simulink library "inp"  (Simulink 8.2)
settings: Solver=ode45  SolverMode=SingleTasking  StartTime=0.0  StopTime=10.0  RelTol=1e-3
undefined symbols (not defined in the file or in its folder): C_TICK_TIME_S, ENUM_FAULTSTATE_FAIL, ENUM_FAULTSTATE_INDETERMINATE, ENUM_FAULTSTATE_PASS, ENUM_KEYSWITCH_ON, FALSE, KINP_ABS1CANCommuOk_flg_ovrdflg, KINP_ABS1CANCommuOk_flg_ovrdval, KINP_ABS1CANPeriodTime_s, KINP_ABS1CANRolCntOk_flg_ovrdflg, KINP_ABS1CANRolCntOk_flg_ovrdval, KINP_ABS2CANChkSumOk_flg_ovrdflg, KINP_ABS2CANChkSumOk_flg_ovrdval, KINP_ABS2CANCommuOk_flg_ovrdflg, KINP_ABS2CANCommuOk_flg_ovrdval, KINP_ABS2CANPeriodTime_s, KINP_ABS2CANRolCntOk_flg_ovrdflg, KINP_ABS2CANRolCntOk_flg_ovrdval, KINP_ABS3CANCommuOk_flg_ovrdflg, KINP_ABS3CANCommuOk_flg_ovrdval, KINP_ABS4CANCommuOk_flg_ovrdflg, KINP_ABS4CANCommuOk_flg_ovrdval, KINP_ABSActive_flg_defval, KINP_ABSActive_flg_invalid, KINP_ABSActive_flg_ovrdflg, KINP_ABSActive_flg_ovrdval, KINP_ABSActive_flg_setvalid, KINP_ABSActive_flg_srvcflg, KINP_ABSActive_flg_srvcval, KINP_ABSCANAlive_flg_ovrdflg, KINP_ABSCANAlive_flg_ovrdval, KINP_ABSCANCommuOk_flg_ovrdflg, KINP_ABSCANCommuOk_flg_ovrdval, KINP_ABSCANValidOk_flg_ovrdflg, KINP_ABSCANValidOk_flg_ovrdval, KINP_ABSFault_flg_defval, KINP_ABSFault_flg_invalid, KINP_ABSFault_flg_ovrdflg, KINP_ABSFault_flg_ovrdval, KINP_ABSFault_flg_setvalid, KINP_ABSFault_flg_srvcflg, KINP_ABSFault_flg_srvcval, KINP_AC1CANCommuOk_flg_ovrdflg, KINP_AC1CANCommuOk_flg_ovrdval, KINP_ACCANAlive_flg_ovrdflg, KINP_ACCANAlive_flg_ovrdval, KINP_ACCANCommuOk_flg_ovrdflg, KINP_ACCANCommuOk_flg_ovrdval, KINP_ACCANValidOk_flg_ovrdflg, KINP_ACCANValidOk_flg_ovrdval, KINP_ACCCancelH_flg_defval, KINP_ACCCancelH_flg_invalid, KINP_ACCCancelH_flg_ovrdflg, KINP_ACCCancelH_flg_ovrdval, KINP_ACCCancelH_flg_setvalid, KINP_ACCCancelH_flg_srvcflg, KINP_ACCCancelH_flg_srvcval, KINP_AccelPedalPosn_pct_defval, KINP_AccelPedalPosn_pct_gain, KINP_AccelPedalPosn_pct_invalid ... (+1866)
libraries: inp x192, hcu_lib x34, simulink x33
(part 16 of 21)

== system inp/INP/VCU_signals/AckCurPTTqReqApplable ==
  signal_in : Inport
  msg_valid : Inport  Port=2
  Active_fault_flg : Inport  Port=3
  can_signal_process_wio_validity : inp/can_signal_process_wio_validity
  defval : Constant  Value=KINP_AckCurPTTqReqApplable_flg_defval
  invalid : Constant  Value=KINP_AckCurPTTqReqApplable_flg_invalid
  ovrdflg : Constant  Value=KINP_AckCurPTTqReqApplable_flg_ovrdflg
  ovrdval : Constant  Value=KINP_AckCurPTTqReqApplable_flg_ovrdval
  setvalid : Constant  Value=KINP_AckCurPTTqReqApplable_flg_setvalid
  srvcflg : Constant  Value=KINP_AckCurPTTqReqApplable_flg_srvcflg
  srvcval : Constant  Value=KINP_AckCurPTTqReqApplable_flg_srvcval
  signal_out : Outport
  signal_valid_out : Outport  Port=2
  wires:
  can_signal_process_wio_validity/1 -> signal_out/1
  can_signal_process_wio_validity/2 -> signal_valid_out/1
  signal_in/1 -> can_signal_process_wio_validity/1
  msg_valid/1 -> can_signal_process_wio_validity/2
  defval/1 -> can_signal_process_wio_validity/4
  invalid/1 -> can_signal_process_wio_validity/5
  setvalid/1 -> can_signal_process_wio_validity/6
  ovrdflg/1 -> can_signal_process_wio_validity/7
  ovrdval/1 -> can_signal_process_wio_validity/8
  srvcflg/1 -> can_signal_process_wio_validity/9
  srvcval/1 -> can_signal_process_wio_validity/10
  Active_fault_flg/1 -> can_signal_process_wio_validity/3

== system inp/INP/VCU_signals/AckCurVehDec ==
  signal_in : Inport
  signal_valid_in : Inport  Port=2
  msg_valid : Inport  Port=3
  Active_fault_flg : Inport  Port=4
  can_signal_process_with_validity : inp/can_signal_process_with_validity
  conv_gain_offset : Subsystem
  defval : Constant  Value=KINP_AckCurVehDec_mps2_defval
  invalid : Constant  Value=KINP_AckCurVehDec_mps2_invalid
  min_max_limit : Subsystem
  ovrdflg : Constant  Value=KINP_AckCurVehDec_mps2_ovrdflg
  ovrdval : Constant  Value=KINP_AckCurVehDec_mps2_ovrdval
  setvalid : Constant  Value=KINP_AckCurVehDec_mps2_setvalid
  srvcflg : Constant  Value=KINP_AckCurVehDec_mps2_srvcflg
  srvcval : Constant  Value=KINP_AckCurVehDec_mps2_srvcval
  signal_out : Outport
  signal_valid_out : Outport  Port=2
  raw_signal_valid_out : Outport  Port=3
  wires:
  can_signal_process_with_validity/1 -> min_max_limit/1(in)
  can_signal_process_with_validity/2 -> signal_valid_out/1
  signal_in/1 -> conv_gain_offset/1(in)
  signal_valid_in/1 -> can_signal_process_with_validity/2
  msg_valid/1 -> can_signal_process_with_validity/3, can_signal_process_with_validity/5
  defval/1 -> can_signal_process_with_validity/6
  invalid/1 -> can_signal_process_with_validity/7
  conv_gain_offset/1(out) -> can_signal_process_with_validity/1
  min_max_limit/1(out) -> signal_out/1
  setvalid/1 -> can_signal_process_with_validity/8
  ovrdflg/1 -> can_signal_process_with_validity/9
  ovrdval/1 -> can_signal_process_with_validity/10
  srvcflg/1 -> can_signal_process_with_validity/11
  srvcval/1 -> can_signal_process_with_validity/12
  Active_fault_flg/1 -> can_signal_process_with_validity/4
  can_signal_process_with_validity/3 -> raw_signal_valid_out/1

== system inp/INP/VCU_signals/CurDriverTqReq ==
  signal_in : Inport
  signal_valid_in : Inport  Port=2
  msg_valid : Inport  Port=3
  Active_fault_flg : Inport  Port=4
  can_signal_process_with_validity : inp/can_signal_process_with_validity
  conv_gain_offset : Subsystem
  defval : Constant  Value=KINP_CurDriverTqReq_Nm_defval
  invalid : Constant  Value=KINP_CurDriverTqReq_Nm_invalid
  min_max_limit : Subsystem
  ovrdflg : Constant  Value=KINP_CurDriverTqReq_Nm_ovrdflg
  ovrdval : Constant  Value=KINP_CurDriverTqReq_Nm_ovrdval
  setvalid : Constant  Value=KINP_CurDriverTqReq_Nm_setvalid
  srvcflg : Constant  Value=KINP_CurDriverTqReq_Nm_srvcflg
  srvcval : Constant  Value=KINP_CurDriverTqReq_Nm_srvcval
  signal_out : Outport
  signal_valid_out : Outport  Port=2
  raw_signal_valid_out : Outport  Port=3
  wires:
  can_signal_process_with_validity/3 -> raw_signal_valid_out/1
  Active_fault_flg/1 -> can_signal_process_with_validity/4
  srvcval/1 -> can_signal_process_with_validity/12
  srvcflg/1 -> can_signal_process_with_validity/11
  ovrdval/1 -> can_signal_process_with_validity/10
  ovrdflg/1 -> can_signal_process_with_validity/9
  setvalid/1 -> can_signal_process_with_validity/8
  min_max_limit/1(out) -> signal_out/1
  conv_gain_offset/1(out) -> can_signal_process_with_validity/1
  invalid/1 -> can_signal_process_with_validity/7
  defval/1 -> can_signal_process_with_validity/6
  msg_valid/1 -> can_signal_process_with_validity/5, can_signal_process_with_validity/3
  signal_valid_in/1 -> can_signal_process_with_validity/2
  signal_in/1 -> conv_gain_offset/1(in)
  can_signal_process_with_validity/2 -> signal_valid_out/1
  can_signal_process_with_validity/1 -> min_max_limit/1(in)

== system inp/INP/VCU_signals/DeceContManag ==
  signal_in : Inport
  msg_valid : Inport  Port=2
  Active_fault_flg : Inport  Port=3
  can_signal_process_wio_validity : inp/can_signal_process_wio_validity
  defval : Constant  Value=KINP_DeceContManag_flg_defval
  invalid : Constant  Value=KINP_DeceContManag_flg_invalid
  ovrdflg : Constant  Value=KINP_DeceContManag_flg_ovrdflg
  ovrdval : Constant  Value=KINP_DeceContManag_flg_ovrdval
  setvalid : Constant  Value=KINP_DeceContManag_flg_setvalid
  srvcflg : Constant  Value=KINP_DeceContManag_flg_srvcflg
  srvcval : Constant  Value=KINP_DeceContManag_flg_srvcval
  signal_out : Outport
  signal_valid_out : Outport  Port=2
  wires:
  can_signal_process_wio_validity/1 -> signal_out/1
  can_signal_process_wio_validity/2 -> signal_valid_out/1
  signal_in/1 -> can_signal_process_wio_validity/1
  msg_valid/1 -> can_signal_process_wio_validity/2
  defval/1 -> can_signal_process_wio_validity/4
  invalid/1 -> can_signal_process_wio_validity/5
  setvalid/1 -> can_signal_process_wio_validity/6
  ovrdflg/1 -> can_signal_process_wio_validity/7
  ovrdval/1 -> can_signal_process_wio_validity/8
  srvcflg/1 -> can_signal_process_wio_validity/9
  srvcval/1 -> can_signal_process_wio_validity/10
  Active_fault_flg/1 -> can_signal_process_wio_validity/3

== system inp/INP/VCU_signals/DriverBrakPedalOn ==
  signal_in : Inport
  signal_valid_in : Inport  Port=2
  msg_valid : Inport  Port=3
  Active_fault_flg : Inport  Port=4
  can_signal_process_with_validity : inp/can_signal_process_with_validity
  defval : Constant  Value=KINP_DriverBrakPedalOn_flg_defval
  invalid : Constant  Value=KINP_DriverBrakPedalOn_flg_invalid
  ovrdflg : Constant  Value=KINP_DriverBrakPedalOn_flg_ovrdflg
  ovrdval : Constant  Value=KINP_DriverBrakPedalOn_flg_ovrdval
  setvalid : Constant  Value=KINP_DriverBrakPedalOn_flg_setvalid
  srvcflg : Constant  Value=KINP_DriverBrakPedalOn_flg_srvcflg
  srvcval : Constant  Value=KINP_DriverBrakPedalOn_flg_srvcval
  signal_out : Outport
  signal_valid_out : Outport  Port=2
  raw_signal_valid_out : Outport  Port=3
  wires:
  Active_fault_flg/1 -> can_signal_process_with_validity/4
  srvcval/1 -> can_signal_process_with_validity/12
  srvcflg/1 -> can_signal_process_with_validity/11
  ovrdval/1 -> can_signal_process_with_validity/10
  ovrdflg/1 -> can_signal_process_with_validity/9
  setvalid/1 -> can_signal_process_with_validity/8
  invalid/1 -> can_signal_process_with_validity/7
  defval/1 -> can_signal_process_with_validity/6
  msg_valid/1 -> can_signal_process_with_validity/3, can_signal_process_with_validity/5
  signal_valid_in/1 -> can_signal_process_with_validity/2
  signal_in/1 -> can_signal_process_with_validity/1
  can_signal_process_with_validity/2 -> signal_valid_out/1
  can_signal_process_with_validity/1 -> signal_out/1
  can_signal_process_with_validity/3 -> raw_signal_valid_out/1

== system inp/INP/VCU_signals/ECMExtnTqAvailable ==
  signal_in : Inport
  msg_valid : Inport  Port=2
  Active_fault_flg : Inport  Port=3
  can_signal_process_wio_validity : inp/can_signal_process_wio_validity
  defval : Constant  Value=KINP_ECMExtnTqAvailable_flg_defval
  invalid : Constant  Value=KINP_ECMExtnTqAvailable_flg_invalid
  ovrdflg : Constant  Value=KINP_ECMExtnTqAvailable_flg_ovrdflg
  ovrdval : Constant  Value=KINP_ECMExtnTqAvailable_flg_ovrdval
  setvalid : Constant  Value=KINP_ECMExtnTqAvailable_flg_setvalid
  srvcflg : Constant  Value=KINP_ECMExtnTqAvailable_flg_srvcflg
  srvcval : Constant  Value=KINP_ECMExtnTqAvailable_flg_srvcval
  signal_out : Outport
  signal_valid_out : Outport  Port=2
  wires:
  can_signal_process_wio_validity/1 -> signal_out/1
  can_signal_process_wio_validity/2 -> signal_valid_out/1
  signal_in/1 -> can_signal_process_wio_validity/1
  msg_valid/1 -> can_signal_process_wio_validity/2
  defval/1 -> can_signal_process_wio_validity/4
  invalid/1 -> can_signal_process_wio_validity/5
  setvalid/1 -> can_signal_process_wio_validity/6
  ovrdflg/1 -> can_signal_process_wio_validity/7
  ovrdval/1 -> can_signal_process_wio_validity/8
  srvcflg/1 -> can_signal_process_wio_validity/9
  srvcval/1 -> can_signal_process_wio_validity/10
  Active_fault_flg/1 -> can_signal_process_wio_validity/3

== system inp/INP/VCU_signals/ECMTqSource ==
  signal_in : Inport
  msg_valid : Inport  Port=2
  Active_fault_flg : Inport  Port=3
  can_signal_process_wio_validity : inp/can_signal_process_wio_validity
  defval : Constant  Value=KINP_ECMTqSource_enum_defval
  invalid : Constant  Value=KINP_ECMTqSource_enum_invalid
  ovrdflg : Constant  Value=KINP_ECMTqSource_enum_ovrdflg
  ovrdval : Constant  Value=KINP_ECMTqSource_enum_ovrdval
  setvalid : Constant  Value=KINP_ECMTqSource_enum_setvalid
  srvcflg : Constant  Value=KINP_ECMTqSource_enum_srvcflg
  srvcval : Constant  Value=KINP_ECMTqSource_enum_srvcval
  signal_out : Outport
  signal_valid_out : Outport  Port=2
  wires:
  Active_fault_flg/1 -> can_signal_process_wio_validity/3
  srvcval/1 -> can_signal_process_wio_validity/10
  srvcflg/1 -> can_signal_process_wio_validity/9
  ovrdval/1 -> can_signal_process_wio_validity/8
  ovrdflg/1 -> can_signal_process_wio_validity/7
  setvalid/1 -> can_signal_process_wio_validity/6
  invalid/1 -> can_signal_process_wio_validity/5
  defval/1 -> can_signal_process_wio_validity/4
  msg_valid/1 -> can_signal_process_wio_validity/2
  signal_in/1 -> can_signal_process_wio_validity/1
  can_signal_process_wio_validity/2 -> signal_valid_out/1
  can_signal_process_wio_validity/1 -> signal_out/1

== system inp/INP/VCU_signals/ESCBattVolt ==
  signal_in : Inport
  signal_valid_in : Inport  Port=2
  msg_valid : Inport  Port=3
  Active_fault_flg : Inport  Port=4
  can_signal_process_with_validity : inp/can_signal_process_with_validity
  conv_gain_offset : Subsystem
  defval : Constant  Value=KINP_ESCBattVolt_V_defval
  invalid : Constant  Value=KINP_ESCBattVolt_V_invalid
  min_max_limit : Subsystem
  ovrdflg : Constant  Value=KINP_ESCBattVolt_V_ovrdflg
  ovrdval : Constant  Value=KINP_ESCBattVolt_V_ovrdval
  setvalid : Constant  Value=KINP_ESCBattVolt_V_setvalid
  srvcflg : Constant  Value=KINP_ESCBattVolt_V_srvcflg
  srvcval : Constant  Value=KINP_ESCBattVolt_V_srvcval
  signal_out : Outport
  signal_valid_out : Outport  Port=2
  raw_signal_valid_out : Outport  Port=3
  wires:
  can_signal_process_with_validity/3 -> raw_signal_valid_out/1
  Active_fault_flg/1 -> can_signal_process_with_validity/4
  srvcval/1 -> can_signal_process_with_validity/12
  srvcflg/1 -> can_signal_process_with_validity/11
  ovrdval/1 -> can_signal_process_with_validity/10
  ovrdflg/1 -> can_signal_process_with_validity/9
  setvalid/1 -> can_signal_process_with_validity/8
  min_max_limit/1(out) -> signal_out/1
  conv_gain_offset/1(out) -> can_signal_process_with_validity/1
  invalid/1 -> can_signal_process_with_validity/7
  defval/1 -> can_signal_process_with_validity/6
  msg_valid/1 -> can_signal_process_with_validity/5, can_signal_process_with_validity/3
  signal_valid_in/1 -> can_signal_process_with_validity/2
  signal_in/1 -> conv_gain_offset/1(in)
  can_signal_process_with_validity/2 -> signal_valid_out/1
  can_signal_process_with_validity/1 -> min_max_limit/1(in)

== system inp/INP/VCU_signals/ESCBrakeStatus ==
  signal_in : Inport
  msg_valid : Inport  Port=2
  Active_fault_flg : Inport  Port=3
  can_signal_process_wio_validity : inp/can_signal_process_wio_validity
  defval : Constant  Value=KINP_ESCBrakeStatus_enum_defval
  invalid : Constant  Value=KINP_ESCBrakeStatus_enum_invalid
  ovrdflg : Constant  Value=KINP_ESCBrakeStatus_enum_ovrdflg
  ovrdval : Constant  Value=KINP_ESCBrakeStatus_enum_ovrdval
  setvalid : Constant  Value=KINP_ESCBrakeStatus_enum_setvalid
  srvcflg : Constant  Value=KINP_ESCBrakeStatus_enum_srvcflg
  srvcval : Constant  Value=KINP_ESCBrakeStatus_enum_srvcval
  signal_out : Outport
  signal_valid_out : Outport  Port=2
  wires:
  can_signal_process_wio_validity/1 -> signal_out/1
  can_signal_process_wio_validity/2 -> signal_valid_out/1
  signal_in/1 -> can_signal_process_wio_validity/1
  msg_valid/1 -> can_signal_process_wio_validity/2
  defval/1 -> can_signal_process_wio_validity/4
  invalid/1 -> can_signal_process_wio_validity/5
  setvalid/1 -> can_signal_process_wio_validity/6
  ovrdflg/1 -> can_signal_process_wio_validity/7
  ovrdval/1 -> can_signal_process_wio_validity/8
  srvcflg/1 -> can_signal_process_wio_validity/9
  srvcval/1 -> can_signal_process_wio_validity/10
  Active_fault_flg/1 -> can_signal_process_wio_validity/3

== system inp/INP/VCU_signals/ESCExtnBrkAvailable ==
  signal_in : Inport
  msg_valid : Inport  Port=2
  Active_fault_flg : Inport  Port=3
  can_signal_process_wio_validity : inp/can_signal_process_wio_validity
  defval : Constant  Value=KINP_ESCExtnBrkAvailable_flg_defval
  invalid : Constant  Value=KINP_ESCExtnBrkAvailable_flg_invalid
  ovrdflg : Constant  Value=KINP_ESCExtnBrkAvailable_flg_ovrdflg
  ovrdval : Constant  Value=KINP_ESCExtnBrkAvailable_flg_ovrdval
  setvalid : Constant  Value=KINP_ESCExtnBrkAvailable_flg_setvalid
  srvcflg : Constant  Value=KINP_ESCExtnBrkAvailable_flg_srvcflg
  srvcval : Constant  Value=KINP_ESCExtnBrkAvailable_flg_srvcval
  signal_out : Outport
  signal_valid_out : Outport  Port=2
  wires:
  Active_fault_flg/1 -> can_signal_process_wio_validity/3
  srvcval/1 -> can_signal_process_wio_validity/10
  srvcflg/1 -> can_signal_process_wio_validity/9
  ovrdval/1 -> can_signal_process_wio_validity/8
  ovrdflg/1 -> can_signal_process_wio_validity/7
  setvalid/1 -> can_signal_process_wio_validity/6
  invalid/1 -> can_signal_process_wio_validity/5
  defval/1 -> can_signal_process_wio_validity/4
  msg_valid/1 -> can_signal_process_wio_validity/2
  signal_in/1 -> can_signal_process_wio_validity/1
  can_signal_process_wio_validity/2 -> signal_valid_out/1
  can_signal_process_wio_validity/1 -> signal_out/1

== system inp/INP/VCU_signals/HCUMILReq ==
  signal_in : Inport
  msg_valid : Inport  Port=2
  Active_fault_flg : Inport  Port=3
  can_signal_process_wio_validity : inp/can_signal_process_wio_validity
  defval : Constant  Value=KINP_HCUMILReq_flg_defval
  invalid : Constant  Value=KINP_HCUMILReq_flg_invalid
  ovrdflg : Constant  Value=KINP_HCUMILReq_flg_ovrdflg
  ovrdval : Constant  Value=KINP_HCUMILReq_flg_ovrdval
  setvalid : Constant  Value=KINP_HCUMILReq_flg_setvalid
  srvcflg : Constant  Value=KINP_HCUMILReq_flg_srvcflg
  srvcval : Constant  Value=KINP_HCUMILReq_flg_srvcval
  signal_out : Outport
  signal_valid_out : Outport  Port=2
  wires:
  Active_fault_flg/1 -> can_signal_process_wio_validity/3
  srvcval/1 -> can_signal_process_wio_validity/10
  srvcflg/1 -> can_signal_process_wio_validity/9
  ovrdval/1 -> can_signal_process_wio_validity/8
  ovrdflg/1 -> can_signal_process_wio_validity/7
  setvalid/1 -> can_signal_process_wio_validity/6
  invalid/1 -> can_signal_process_wio_validity/5
  defval/1 -> can_signal_process_wio_validity/4
  msg_valid/1 -> can_signal_process_wio_validity/2
  signal_in/1 -> can_signal_process_wio_validity/1
  can_signal_process_wio_validity/2 -> signal_valid_out/1
  can_signal_process_wio_validity/1 -> signal_out/1

== system inp/INP/VCU_signals/HWAccelPedalPosn ==
  signal_in : Inport
  signal_valid_in : Inport  Port=2
  msg_valid : Inport  Port=3
  Active_fault_flg : Inport  Port=4
  can_signal_process_with_validity : inp/can_signal_process_with_validity
  conv_gain_offset : Subsystem
  defval : Constant  Value=KINP_HWAccelPedalPosn_pct_defval
  invalid : Constant  Value=KINP_HWAccelPedalPosn_pct_invalid
  min_max_limit : Subsystem
  ovrdflg : Constant  Value=KINP_HWAccelPedalPosn_pct_ovrdflg
  ovrdval : Constant  Value=KINP_HWAccelPedalPosn_pct_ovrdval
  setvalid : Constant  Value=KINP_HWAccelPedalPosn_pct_setvalid
  srvcflg : Constant  Value=KINP_HWAccelPedalPosn_pct_srvcflg
  srvcval : Constant  Value=KINP_HWAccelPedalPosn_pct_srvcval
  signal_out : Outport
  signal_valid_out : Outport  Port=2
  raw_signal_valid_out : Outport  Port=3
  wires:
  can_signal_process_with_validity/1 -> min_max_limit/1(in)
  can_signal_process_with_validity/2 -> signal_valid_out/1
  signal_in/1 -> conv_gain_offset/1(in)
  signal_valid_in/1 -> can_signal_process_with_validity/2
  msg_valid/1 -> can_signal_process_with_validity/3, can_signal_process_with_validity/5
  defval/1 -> can_signal_process_with_validity/6
  invalid/1 -> can_signal_process_with_validity/7
  conv_gain_offset/1(out) -> can_signal_process_with_validity/1
  min_max_limit/1(out) -> signal_out/1
  setvalid/1 -> can_signal_process_with_validity/8
  ovrdflg/1 -> can_signal_process_with_validity/9
  ovrdval/1 -> can_signal_process_with_validity/10
  srvcflg/1 -> can_signal_process_with_validity/11
  srvcval/1 -> can_signal_process_with_validity/12
  Active_fault_flg/1 -> can_signal_process_with_validity/4
  can_signal_process_with_validity/3 -> raw_signal_valid_out/1

== system inp/INP/VCU_signals/LFCOnVCU ==
  signal_in : Inport
  msg_valid : Inport  Port=2
  Active_fault_flg : Inport  Port=3
  can_signal_process_wio_validity : inp/can_signal_process_wio_validity
  defval : Constant  Value=KINP_LFCOnVCU_flg_defval
  invalid : Constant  Value=KINP_LFCOnVCU_flg_invalid
  ovrdflg : Constant  Value=KINP_LFCOnVCU_flg_ovrdflg
  ovrdval : Constant  Value=KINP_LFCOnVCU_flg_ovrdval
  setvalid : Constant  Value=KINP_LFCOnVCU_flg_setvalid
  srvcflg : Constant  Value=KINP_LFCOnVCU_flg_srvcflg
  srvcval : Constant  Value=KINP_LFCOnVCU_flg_srvcval
  signal_out : Outport
  signal_valid_out : Outport  Port=2
  wires:
  Active_fault_flg/1 -> can_signal_process_wio_validity/3
  srvcval/1 -> can_signal_process_wio_validity/10
  srvcflg/1 -> can_signal_process_wio_validity/9
  ovrdval/1 -> can_signal_process_wio_validity/8
  ovrdflg/1 -> can_signal_process_wio_validity/7
  setvalid/1 -> can_signal_process_wio_validity/6
  invalid/1 -> can_signal_process_wio_validity/5
  defval/1 -> can_signal_process_wio_validity/4
  msg_valid/1 -> can_signal_process_wio_validity/2
  signal_in/1 -> can_signal_process_wio_validity/1
  can_signal_process_wio_validity/2 -> signal_valid_out/1
  can_signal_process_wio_validity/1 -> signal_out/1

== system inp/INP/VCU_signals/MSAOnVCU ==
  signal_in : Inport
  msg_valid : Inport  Port=2
  Active_fault_flg : Inport  Port=3
  can_signal_process_wio_validity : inp/can_signal_process_wio_validity
  defval : Constant  Value=KINP_MSAOnVCU_flg_defval
  invalid : Constant  Value=KINP_MSAOnVCU_flg_invalid
  ovrdflg : Constant  Value=KINP_MSAOnVCU_flg_ovrdflg
  ovrdval : Constant  Value=KINP_MSAOnVCU_flg_ovrdval
  setvalid : Constant  Value=KINP_MSAOnVCU_flg_setvalid
  srvcflg : Constant  Value=KINP_MSAOnVCU_flg_srvcflg
  srvcval : Constant  Value=KINP_MSAOnVCU_flg_srvcval
  signal_out : Outport
  signal_valid_out : Outport  Port=2
  wires:
  can_signal_process_wio_validity/1 -> signal_out/1
  can_signal_process_wio_validity/2 -> signal_valid_out/1
  signal_in/1 -> can_signal_process_wio_validity/1
  msg_valid/1 -> can_signal_process_wio_validity/2
  defval/1 -> can_signal_process_wio_validity/4
  invalid/1 -> can_signal_process_wio_validity/5
  setvalid/1 -> can_signal_process_wio_validity/6
  ovrdflg/1 -> can_signal_process_wio_validity/7
  ovrdval/1 -> can_signal_process_wio_validity/8
  srvcflg/1 -> can_signal_process_wio_validity/9
  srvcval/1 -> can_signal_process_wio_validity/10
  Active_fault_flg/1 -> can_signal_process_wio_validity/3

== system inp/INP/ABS_signals/BrakBoosterPres/conv_gain_offset ==
  in : Inport
  Product : Product  RndMeth=Zero
  Sum : Sum
  gain : Constant  Value=KINP_BrakBoosterPres_kPa_gain
  offset : Constant  Value=KINP_BrakBoosterPres_kPa_offset
  out : Outport
  wires:
  offset/1 -> Sum/2
  Product/1 -> Sum/1
  gain/1 -> Product/2
  in/1 -> Product/1
  Sum/1 -> out/1

== system inp/INP/ABS_signals/BrakBoosterPres/min_max_limit ==
  in : Inport
  MinMax10 : MinMax  Function=max  Inputs=2
  MinMax5 : MinMax  Inputs=2
  maxval : Constant  Value=KINP_BrakBoosterPres_kPa_maxval
  minval : Constant  Value=KINP_BrakBoosterPres_kPa_minval
  out : Outport
  wires:
  minval/1 -> MinMax10/2
  maxval/1 -> MinMax5/2
  MinMax10/1 -> out/1
  in/1 -> MinMax5/1
  MinMax5/1 -> MinMax10/1

== system inp/INP/ABS_signals/LFWheelSpeed/conv_gain_offset ==
  in : Inport
  Product : Product  RndMeth=Zero
  Sum : Sum
  gain : Constant  Value=KINP_LFWheelSpeed_kph_gain
  offset : Constant  Value=KINP_LFWheelSpeed_kph_offset
  out : Outport
  wires:
  offset/1 -> Sum/2
  Product/1 -> Sum/1
  gain/1 -> Product/2
  in/1 -> Product/1
  Sum/1 -> out/1

== system inp/INP/ABS_signals/LFWheelSpeed/min_max_limit ==
  in : Inport
  MinMax10 : MinMax  Function=max  Inputs=2
  MinMax5 : MinMax  Inputs=2
  maxval : Constant  Value=KINP_LFWheelSpeed_kph_maxval
  minval : Constant  Value=KINP_LFWheelSpeed_kph_minval
  out : Outport
  wires:
  minval/1 -> MinMax10/2
  maxval/1 -> MinMax5/2
  MinMax10/1 -> out/1
  in/1 -> MinMax5/1
  MinMax5/1 -> MinMax10/1

== system inp/INP/ABS_signals/LRWheelSpeed/conv_gain_offset ==
  in : Inport
  Product : Product  RndMeth=Zero
  Sum : Sum
  gain : Constant  Value=KINP_LRWheelSpeed_kph_gain
  offset : Constant  Value=KINP_LRWheelSpeed_kph_offset
  out : Outport
  wires:
  Sum/1 -> out/1
  in/1 -> Product/1
  gain/1 -> Product/2
  Product/1 -> Sum/1
  offset/1 -> Sum/2

== system inp/INP/ABS_signals/LRWheelSpeed/min_max_limit ==
  in : Inport
  MinMax10 : MinMax  Function=max  Inputs=2
  MinMax5 : MinMax  Inputs=2
  maxval : Constant  Value=KINP_LRWheelSpeed_kph_maxval
  minval : Constant  Value=KINP_LRWheelSpeed_kph_minval
  out : Outport
  wires:
  MinMax5/1 -> MinMax10/1
  in/1 -> MinMax5/1
  MinMax10/1 -> out/1
  maxval/1 -> MinMax5/2
  minval/1 -> MinMax10/2

== system inp/INP/ABS_signals/LatAccel/conv_gain_offset ==
  in : Inport
  Product : Product  RndMeth=Zero
  Sum : Sum
  gain : Constant  Value=KINP_LatAccel_G_gain
  offset : Constant  Value=KINP_LatAccel_G_offset
  out : Outport
  wires:
  offset/1 -> Sum/2
  Product/1 -> Sum/1
  gain/1 -> Product/2
  in/1 -> Product/1
  Sum/1 -> out/1

== system inp/INP/ABS_signals/LatAccel/min_max_limit ==
  in : Inport
  MinMax10 : MinMax  Function=max  Inputs=2
  MinMax5 : MinMax  Inputs=2
  maxval : Constant  Value=KINP_LatAccel_G_maxval
  minval : Constant  Value=KINP_LatAccel_G_minval
  out : Outport
  wires:
  minval/1 -> MinMax10/2
  maxval/1 -> MinMax5/2
  MinMax10/1 -> out/1
  in/1 -> MinMax5/1
  MinMax5/1 -> MinMax10/1

== system inp/INP/ABS_signals/LongAccel/conv_gain_offset ==
  in : Inport
  Product : Product  RndMeth=Zero
  Sum : Sum
  gain : Constant  Value=KINP_LongAccel_G_gain
  offset : Constant  Value=KINP_LongAccel_G_offset
  out : Outport
  wires:
  Sum/1 -> out/1
  in/1 -> Product/1
  gain/1 -> Product/2
  Product/1 -> Sum/1
  offset/1 -> Sum/2

== system inp/INP/ABS_signals/LongAccel/min_max_limit ==
  in : Inport
  MinMax10 : MinMax  Function=max  Inputs=2
  MinMax5 : MinMax  Inputs=2
  maxval : Constant  Value=KINP_LongAccel_G_maxval
  minval : Constant  Value=KINP_LongAccel_G_minval
  out : Outport
  wires:
  MinMax5/1 -> MinMax10/1
  in/1 -> MinMax5/1
  MinMax10/1 -> out/1
  maxval/1 -> MinMax5/2
  minval/1 -> MinMax10/2

== system inp/INP/ABS_signals/MCPressure/conv_gain_offset ==
  in : Inport
  Product : Product  RndMeth=Zero
  Sum : Sum
  gain : Constant  Value=KINP_MCPressure_bar_gain
  offset : Constant  Value=KINP_MCPressure_bar_offset
  out : Outport
  wires:
  offset/1 -> Sum/2
  Product/1 -> Sum/1
  gain/1 -> Product/2
  in/1 -> Product/1
  Sum/1 -> out/1

== system inp/INP/ABS_signals/MCPressure/min_max_limit ==
  in : Inport
  MinMax10 : MinMax  Function=max  Inputs=2
  MinMax5 : MinMax  Inputs=2
  maxval : Constant  Value=KINP_MCPressure_bar_maxval
  minval : Constant  Value=KINP_MCPressure_bar_minval
  out : Outport
  wires:
  minval/1 -> MinMax10/2
  maxval/1 -> MinMax5/2
  MinMax10/1 -> out/1
  in/1 -> MinMax5/1
  MinMax5/1 -> MinMax10/1

== system inp/INP/ABS_signals/RFWheelSpeed/conv_gain_offset ==
  in : Inport
  Product : Product  RndMeth=Zero
  Sum : Sum
  gain : Constant  Value=KINP_RFWheelSpeed_kph_gain
  offset : Constant  Value=KINP_RFWheelSpeed_kph_offset
  out : Outport
  wires:
  offset/1 -> Sum/2
  Product/1 -> Sum/1
  gain/1 -> Product/2
  in/1 -> Product/1
  Sum/1 -> out/1

== system inp/INP/ABS_signals/RFWheelSpeed/min_max_limit ==
  in : Inport
  MinMax10 : MinMax  Function=max  Inputs=2
  MinMax5 : MinMax  Inputs=2
  maxval : Constant  Value=KINP_RFWheelSpeed_kph_maxval
  minval : Constant  Value=KINP_RFWheelSpeed_kph_minval
  out : Outport
  wires:
  minval/1 -> MinMax10/2
  maxval/1 -> MinMax5/2
  MinMax10/1 -> out/1
  in/1 -> MinMax5/1
  MinMax5/1 -> MinMax10/1

== system inp/INP/ABS_signals/RRWheelSpeed/conv_gain_offset ==
  in : Inport
  Product : Product  RndMeth=Zero
  Sum : Sum
  gain : Constant  Value=KINP_RRWheelSpeed_kph_gain
  offset : Constant  Value=KINP_RRWheelSpeed_kph_offset
  out : Outport
  wires:
  Sum/1 -> out/1
  in/1 -> Product/1
  gain/1 -> Product/2
  Product/1 -> Sum/1
  offset/1 -> Sum/2

== system inp/INP/ABS_signals/RRWheelSpeed/min_max_limit ==
  in : Inport
  MinMax10 : MinMax  Function=max  Inputs=2
  MinMax5 : MinMax  Inputs=2
  maxval : Constant  Value=KINP_RRWheelSpeed_kph_maxval
  minval : Constant  Value=KINP_RRWheelSpeed_kph_minval
  out : Outport
  wires:
  MinMax5/1 -> MinMax10/1
  in/1 -> MinMax5/1
  MinMax10/1 -> out/1
  maxval/1 -> MinMax5/2
  minval/1 -> MinMax10/2

== system inp/INP/ABS_signals/SurfaceGrade/conv_gain_offset ==
  in : Inport
  Product : Product  RndMeth=Zero
  Sum : Sum
  gain : Constant  Value=KINP_SurfaceGrade_deg_gain
  offset : Constant  Value=KINP_SurfaceGrade_deg_offset
  out : Outport
  wires:
  Sum/1 -> out/1
  in/1 -> Product/1
  gain/1 -> Product/2
  Product/1 -> Sum/1
  offset/1 -> Sum/2

== system inp/INP/ABS_signals/SurfaceGrade/min_max_limit ==
  in : Inport
  MinMax10 : MinMax  Function=max  Inputs=2
  MinMax5 : MinMax  Inputs=2
  maxval : Constant  Value=KINP_SurfaceGrade_deg_maxval
  minval : Constant  Value=KINP_SurfaceGrade_deg_minval
  out : Outport
  wires:
  MinMax5/1 -> MinMax10/1
  in/1 -> MinMax5/1
  MinMax10/1 -> out/1
  maxval/1 -> MinMax5/2
  minval/1 -> MinMax10/2

== system inp/INP/ABS_signals/VehicleSpeed/conv_gain_offset ==
  in : Inport
  Product : Product  RndMeth=Zero
  Sum : Sum
  gain : Constant  Value=KINP_VehSpd_kph_gain
  offset : Constant  Value=KINP_VehSpd_kph_offset
  out : Outport
  wires:
  Sum/1 -> out/1
  in/1 -> Product/1
  gain/1 -> Product/2
  Product/1 -> Sum/1
  offset/1 -> Sum/2

== system inp/INP/ABS_signals/VehicleSpeed/min_max_limit ==
  in : Inport
  MinMax10 : MinMax  Function=max  Inputs=2
  MinMax5 : MinMax  Inputs=2
  maxval : Constant  Value=KINP_VehSpd_kph_maxval
  minval : Constant  Value=KINP_VehSpd_kph_minval
  out : Outport
  wires:
  MinMax5/1 -> MinMax10/1
  in/1 -> MinMax5/1
  MinMax10/1 -> out/1
  maxval/1 -> MinMax5/2
  minval/1 -> MinMax10/2

== system inp/INP/ABS_signals/YawRate/conv_gain_offset ==
  in : Inport
  Product : Product  RndMeth=Zero
  Sum : Sum
  gain : Constant  Value=KINP_YawRate_degps_gain
  offset : Constant  Value=KINP_YawRate_degps_offset
  out : Outport
  wires:
  Sum/1 -> out/1
  in/1 -> Product/1
  gain/1 -> Product/2
  Product/1 -> Sum/1
  offset/1 -> Sum/2

== system inp/INP/ABS_signals/YawRate/min_max_limit ==
  in : Inport
  MinMax10 : MinMax  Function=max  Inputs=2
  MinMax5 : MinMax  Inputs=2
  maxval : Constant  Value=KINP_YawRate_degps_maxval
  minval : Constant  Value=KINP_YawRate_degps_minval
  out : Outport
  wires:
  MinMax5/1 -> MinMax10/1
  in/1 -> MinMax5/1
  MinMax10/1 -> out/1
  maxval/1 -> MinMax5/2
  minval/1 -> MinMax10/2

== system inp/INP/AC_signals/OutsideTemp/conv_gain_offset ==
  in : Inport
  Product : Product  RndMeth=Zero
  Sum : Sum
  gain : Constant  Value=KINP_OutsideTemp_degC_gain
  offset : Constant  Value=KINP_OutsideTemp_degC_offset
  out : Outport
  wires:
  offset/1 -> Sum/2
  Product/1 -> Sum/1
  gain/1 -> Product/2
  in/1 -> Product/1
  Sum/1 -> out/1

== system inp/INP/AC_signals/OutsideTemp/min_max_limit ==
  in : Inport
  MinMax10 : MinMax  Function=max  Inputs=2
  MinMax5 : MinMax  Inputs=2
  maxval : Constant  Value=KINP_OutsideTemp_degC_maxval
  minval : Constant  Value=KINP_OutsideTemp_degC_minval
  out : Outport
  wires:
  minval/1 -> MinMax10/2
  maxval/1 -> MinMax5/2
  MinMax10/1 -> out/1
  in/1 -> MinMax5/1
  MinMax5/1 -> MinMax10/1

== system inp/INP/CAN_Message_Valid_Proc/EPMValid/EPM1Valid ==
  signal_in : Inport
  Active_fault_flg : Inport  Port=2
  TRUE : Constant  Value=TRUE
  can_valid_process : inp/can_valid_process
  defval : Constant  Value=KINP_CANMsgValidEPM1_flg_defval
  invalid : Constant  Value=KINP_CANMsgValidEPM1_flg_invalid
  ovrdflg : Constant  Value=KINP_CANMsgValidEPM1_flg_ovrdflg
  ovrdval : Constant  Value=KINP_CANMsgValidEPM1_flg_ovrdval
  setvalid : Constant  Value=KINP_CANMsgValidEPM1_flg_setvalid
  srvcflg : Constant  Value=KINP_CANMsgValidEPM1_flg_srvcflg
  srvcval : Constant  Value=KINP_CANMsgValidEPM1_flg_srvcval
  tempval : Constant  Value=KINP_CANMsgValidEPM1_flg_tempval
  signal_out : Outport
  signal_valid_out : Outport  Port=2
  wires:
  can_valid_process/1 -> signal_out/1
  can_valid_process/2 -> signal_valid_out/1
  signal_in/1 -> can_valid_process/1
  Active_fault_flg/1 -> can_valid_process/2
  TRUE/1 -> can_valid_process/3
  defval/1 -> can_valid_process/4
  tempval/1 -> can_valid_process/5
  invalid/1 -> can_valid_process/6
  setvalid/1 -> can_valid_process/7
  ovrdflg/1 -> can_valid_process/8
  ovrdval/1 -> can_valid_process/9
  srvcflg/1 -> can_valid_process/10
  srvcval/1 -> can_valid_process/11

== system inp/INP/CAN_Message_Valid_Proc/EPMValid/EPM2Valid ==
  signal_in : Inport
  Active_fault_flg : Inport  Port=2
  TRUE : Constant  Value=TRUE
  can_valid_process : inp/can_valid_process
  defval : Constant  Value=KINP_CANMsgValidEPM2_flg_defval
  invalid : Constant  Value=KINP_CANMsgValidEPM2_flg_invalid
  ovrdflg : Constant  Value=KINP_CANMsgValidEPM2_flg_ovrdflg
  ovrdval : Constant  Value=KINP_CANMsgValidEPM2_flg_ovrdval
  setvalid : Constant  Value=KINP_CANMsgValidEPM2_flg_setvalid
  srvcflg : Constant  Value=KINP_CANMsgValidEPM2_flg_srvcflg
  srvcval : Constant  Value=KINP_CANMsgValidEPM2_flg_srvcval
  tempval : Constant  Value=KINP_CANMsgValidEPM2_flg_tempval
  signal_out : Outport
  signal_valid_out : Outport  Port=2
  wires:
  Active_fault_flg/1 -> can_valid_process/2
  signal_in/1 -> can_valid_process/1
  can_valid_process/2 -> signal_valid_out/1
  can_valid_process/1 -> signal_out/1
  TRUE/1 -> can_valid_process/3
  defval/1 -> can_valid_process/4
  tempval/1 -> can_valid_process/5
  invalid/1 -> can_valid_process/6
  setvalid/1 -> can_valid_process/7
  ovrdflg/1 -> can_valid_process/8
  ovrdval/1 -> can_valid_process/9
  srvcflg/1 -> can_valid_process/10
  srvcval/1 -> can_valid_process/11

== system inp/INP/CAN_Message_Valid_Proc/EPMValid/EPM3Valid ==
  signal_in : Inport
  Active_fault_flg : Inport  Port=2
  TRUE : Constant  Value=TRUE
  can_valid_process : inp/can_valid_process
  defval : Constant  Value=KINP_CANMsgValidEPM3_flg_defval
  invalid : Constant  Value=KINP_CANMsgValidEPM3_flg_invalid
  ovrdflg : Constant  Value=KINP_CANMsgValidEPM3_flg_ovrdflg
  ovrdval : Constant  Value=KINP_CANMsgValidEPM3_flg_ovrdval
  setvalid : Constant  Value=KINP_CANMsgValidEPM3_flg_setvalid
  srvcflg : Constant  Value=KINP_CANMsgValidEPM3_flg_srvcflg
  srvcval : Constant  Value=KINP_CANMsgValidEPM3_flg_srvcval
  tempval : Constant  Value=KINP_CANMsgValidEPM3_flg_tempval
  signal_out : Outport
  signal_valid_out : Outport  Port=2
  wires:
  can_valid_process/1 -> signal_out/1
  can_valid_process/2 -> signal_valid_out/1
  signal_in/1 -> can_valid_process/1
  Active_fault_flg/1 -> can_valid_process/2
  TRUE/1 -> can_valid_process/3
  defval/1 -> can_valid_process/4
  tempval/1 -> can_valid_process/5
  invalid/1 -> can_valid_process/6
  setvalid/1 -> can_valid_process/7
  ovrdflg/1 -> can_valid_process/8
  ovrdval/1 -> can_valid_process/9
  srvcflg/1 -> can_valid_process/10
  srvcval/1 -> can_valid_process/11

== system inp/INP/CAN_Message_Valid_Proc/EPMValid/EPM4Valid ==
  signal_in : Inport
  Active_fault_flg : Inport  Port=2
  TRUE : Constant  Value=TRUE
  can_valid_process : inp/can_valid_process
  defval : Constant  Value=KINP_CANMsgValidEPM4_flg_defval
  invalid : Constant  Value=KINP_CANMsgValidEPM4_flg_invalid
  ovrdflg : Constant  Value=KINP_CANMsgValidEPM4_flg_ovrdflg
  ovrdval : Constant  Value=KINP_CANMsgValidEPM4_flg_ovrdval
  setvalid : Constant  Value=KINP_CANMsgValidEPM4_flg_setvalid
  srvcflg : Constant  Value=KINP_CANMsgValidEPM4_flg_srvcflg
  srvcval : Constant  Value=KINP_CANMsgValidEPM4_flg_srvcval
  tempval : Constant  Value=KINP_CANMsgValidEPM4_flg_tempval
  signal_out : Outport
  signal_valid_out : Outport  Port=2
  wires:
  Active_fault_flg/1 -> can_valid_process/2
  signal_in/1 -> can_valid_process/1
  can_valid_process/2 -> signal_valid_out/1
  can_valid_process/1 -> signal_out/1
  TRUE/1 -> can_valid_process/3
  defval/1 -> can_valid_process/4
  tempval/1 -> can_valid_process/5
  invalid/1 -> can_valid_process/6
  setvalid/1 -> can_valid_process/7
  ovrdflg/1 -> can_valid_process/8
  ovrdval/1 -> can_valid_process/9
  srvcflg/1 -> can_valid_process/10
  srvcval/1 -> can_valid_process/11

== system inp/INP/CAN_Message_Valid_Proc/EPMValid/EPM5Valid ==
  signal_in : Inport
  Active_fault_flg : Inport  Port=2
  TRUE : Constant  Value=TRUE
  can_valid_process : inp/can_valid_process
  defval : Constant  Value=KINP_CANMsgValidEPM5_flg_defval
  invalid : Constant  Value=KINP_CANMsgValidEPM5_flg_invalid
  ovrdflg : Constant  Value=KINP_CANMsgValidEPM5_flg_ovrdflg
  ovrdval : Constant  Value=KINP_CANMsgValidEPM5_flg_ovrdval
  setvalid : Constant  Value=KINP_CANMsgValidEPM5_flg_setvalid
  srvcflg : Constant  Value=KINP_CANMsgValidEPM5_flg_srvcflg
  srvcval : Constant  Value=KINP_CANMsgValidEPM5_flg_srvcval
  tempval : Constant  Value=KINP_CANMsgValidEPM5_flg_tempval
  signal_out : Outport
  signal_valid_out : Outport  Port=2
  wires:
  srvcval/1 -> can_valid_process/11
  srvcflg/1 -> can_valid_process/10
  ovrdval/1 -> can_valid_process/9
  ovrdflg/1 -> can_valid_process/8
  setvalid/1 -> can_valid_process/7
  invalid/1 -> can_valid_process/6
  tempval/1 -> can_valid_process/5
  defval/1 -> can_valid_process/4
  TRUE/1 -> can_valid_process/3
  can_valid_process/1 -> signal_out/1
  can_valid_process/2 -> signal_valid_out/1
  signal_in/1 -> can_valid_process/1
  Active_fault_flg/1 -> can_valid_process/2

== system inp/INP/ECM_signals/AccelPedalPosn/conv_gain_offset ==
  in : Inport
  Product : Product  RndMeth=Zero
  Sum : Sum
  gain : Constant  Value=KINP_AccelPedalPosn_pct_gain
  offset : Constant  Value=KINP_AccelPedalPosn_pct_offset
  out : Outport
  wires:
  offset/1 -> Sum/2
  Product/1 -> Sum/1
  gain/1 -> Product/2
  in/1 -> Product/1
  Sum/1 -> out/1

== system inp/INP/ECM_signals/AccelPedalPosn/min_max_limit ==
  in : Inport
  MinMax10 : MinMax  Function=max  Inputs=2
  MinMax5 : MinMax  Inputs=2
  maxval : Constant  Value=KINP_AccelPedalPosn_pct_maxval
  minval : Constant  Value=KINP_AccelPedalPosn_pct_minval
  out : Outport
  wires:
  minval/1 -> MinMax10/2
  maxval/1 -> MinMax5/2
  MinMax10/1 -> out/1
  in/1 -> MinMax5/1
  MinMax5/1 -> MinMax10/1

== system inp/INP/ECM_signals/EffAccelPedalPosn/conv_gain_offset ==
  in : Inport
  Product : Product  RndMeth=Zero
  Sum : Sum
  gain : Constant  Value=KINP_EffAccelPedalPosn_pct_gain
  offset : Constant  Value=KINP_EffAccelPedalPosn_pct_offset
  out : Outport
  wires:
  Sum/1 -> out/1
  in/1 -> Product/1
  gain/1 -> Product/2
  Product/1 -> Sum/1
  offset/1 -> Sum/2

== system inp/INP/ECM_signals/EffAccelPedalPosn/min_max_limit ==
  in : Inport
  MinMax10 : MinMax  Function=max  Inputs=2
  MinMax5 : MinMax  Inputs=2
  maxval : Constant  Value=KINP_EffAccelPedalPosn_pct_maxval
  minval : Constant  Value=KINP_EffAccelPedalPosn_pct_minval
  out : Outport
  wires:
  MinMax5/1 -> MinMax10/1
  in/1 -> MinMax5/1
  MinMax10/1 -> out/1
  maxval/1 -> MinMax5/2
  minval/1 -> MinMax10/2

== system inp/INP/ECM_signals/EngineFrictionTorque/conv_gain_offset ==
  in : Inport
  Product : Product  RndMeth=Zero
  Sum : Sum
  gain : Constant  Value=KINP_EngTqFriction_Nm_gain
  offset : Constant  Value=KINP_EngTqFriction_Nm_offset
  out : Outport
  wires:
  Sum/1 -> out/1
  in/1 -> Product/1
  gain/1 -> Product/2
  Product/1 -> Sum/1
  offset/1 -> Sum/2

== system inp/INP/ECM_signals/EngineFrictionTorque/min_max_limit ==
  in : Inport
  MinMax10 : MinMax  Function=max  Inputs=2
  MinMax5 : MinMax  Inputs=2
  maxval : Constant  Value=KINP_EngTqFriction_Nm_maxval
  minval : Constant  Value=KINP_EngTqFriction_Nm_minval
  out : Outport
  wires:
  MinMax5/1 -> MinMax10/1
  in/1 -> MinMax5/1
  MinMax10/1 -> out/1
  maxval/1 -> MinMax5/2
  minval/1 -> MinMax10/2

== system inp/INP/ECM_signals/EngineIndicatedTorque/conv_gain_offset ==
  in : Inport
  Product : Product  RndMeth=Zero
  Sum : Sum
  gain : Constant  Value=KINP_EngTqIndicated_Nm_gain
  offset : Constant  Value=KINP_EngTqIndicated_Nm_offset
  out : Outport
  wires:
  offset/1 -> Sum/2
  Product/1 -> Sum/1
  gain/1 -> Product/2
  in/1 -> Product/1
  Sum/1 -> out/1

== system inp/INP/ECM_signals/EngineIndicatedTorque/min_max_limit ==
  in : Inport
  MinMax10 : MinMax  Function=max  Inputs=2
  MinMax5 : MinMax  Inputs=2
  maxval : Constant  Value=KINP_EngTqIndicated_Nm_maxval
  minval : Constant  Value=KINP_EngTqIndicated_Nm_minval
  out : Outport
  wires:
  minval/1 -> MinMax10/2
  maxval/1 -> MinMax5/2
  MinMax10/1 -> out/1
  in/1 -> MinMax5/1
  MinMax5/1 -> MinMax10/1

== system inp/INP/ECM_signals/EngineIndicatedTorqueCorrected/conv_gain_offset ==
  in : Inport
  Product : Product  RndMeth=Zero
  Sum : Sum
  gain : Constant  Value=KINP_EngTqIndicatedCOR_Nm_gain
  offset : Constant  Value=KINP_EngTqIndicatedCOR_Nm_offset
  out : Outport
  wires:
  Sum/1 -> out/1
  in/1 -> Product/1
  gain/1 -> Product/2
  Product/1 -> Sum/1
  offset/1 -> Sum/2

== system inp/INP/ECM_signals/EngineIndicatedTorqueCorrected/min_max_limit ==
  in : Inport
  MinMax10 : MinMax  Function=max  Inputs=2
  MinMax5 : MinMax  Inputs=2
  maxval : Constant  Value=KINP_EngTqIndicatedCOR_Nm_maxval
  minval : Constant  Value=KINP_EngTqIndicatedCOR_Nm_minval
  out : Outport
  wires:
  MinMax5/1 -> MinMax10/1
  in/1 -> MinMax5/1
  MinMax10/1 -> out/1
  maxval/1 -> MinMax5/2
  minval/1 -> MinMax10/2

== system inp/INP/ECM_signals/EngineSpeed/conv_gain_offset ==
  in : Inport
  Product : Product  RndMeth=Zero
  Sum : Sum
  gain : Constant  Value=KINP_EngSpd_erpm_gain
  offset : Constant  Value=KINP_EngSpd_erpm_offset
  out : Outport
  wires:
  offset/1 -> Sum/2
  Product/1 -> Sum/1
  gain/1 -> Product/2
  in/1 -> Product/1
  Sum/1 -> out/1

== system inp/INP/ECM_signals/EngineSpeed/min_max_limit ==
  in : Inport
  MinMax10 : MinMax  Function=max  Inputs=2
  MinMax5 : MinMax  Inputs=2
  maxval : Constant  Value=KINP_EngSpd_erpm_maxval
  minval : Constant  Value=KINP_EngSpd_erpm_minval
  out : Outport
  wires:
  minval/1 -> MinMax10/2
  maxval/1 -> MinMax5/2
  MinMax10/1 -> out/1
  in/1 -> MinMax5/1
  MinMax5/1 -> MinMax10/1

== system inp/INP/ECM_signals/EngineTorqueMax/conv_gain_offset ==
  in : Inport
  Product : Product  RndMeth=Zero
  Sum : Sum
  gain : Constant  Value=KINP_EngTqMax_Nm_gain
  offset : Constant  Value=KINP_EngTqMax_Nm_offset
  out : Outport
  wires:
  offset/1 -> Sum/2
  Product/1 -> Sum/1
  gain/1 -> Product/2
  in/1 -> Product/1
  Sum/1 -> out/1

== system inp/INP/ECM_signals/EngineTorqueMax/min_max_limit ==
  in : Inport
  MinMax10 : MinMax  Function=max  Inputs=2
  MinMax5 : MinMax  Inputs=2
  maxval : Constant  Value=KINP_EngTqMax_Nm_maxval
  minval : Constant  Value=KINP_EngTqMax_Nm_minval
  out : Outport
  wires:
  minval/1 -> MinMax10/2
  maxval/1 -> MinMax5/2
  MinMax10/1 -> out/1
  in/1 -> MinMax5/1
  MinMax5/1 -> MinMax10/1

== system inp/INP/ECM_signals/EngineTorqueMin/conv_gain_offset ==
  in : Inport
  Product : Product  RndMeth=Zero
  Sum : Sum
  gain : Constant  Value=KINP_EngTqMin_Nm_gain
  offset : Constant  Value=KINP_EngTqMin_Nm_offset
  out : Outport
  wires:
  Sum/1 -> out/1
  in/1 -> Product/1
  gain/1 -> Product/2
  Product/1 -> Sum/1
  offset/1 -> Sum/2

== system inp/INP/ECM_signals/EngineTorqueMin/min_max_limit ==
  in : Inport
  MinMax10 : MinMax  Function=max  Inputs=2
  MinMax5 : MinMax  Inputs=2
  maxval : Constant  Value=KINP_EngTqMin_Nm_maxval
  minval : Constant  Value=KINP_EngTqMin_Nm_minval
  out : Outport
  wires:
  MinMax5/1 -> MinMax10/1
  in/1 -> MinMax5/1
  MinMax10/1 -> out/1
  maxval/1 -> MinMax5/2
  minval/1 -> MinMax10/2

== system inp/INP/EPM_signals/LaneC0Left/conv_gain_offset ==
  in : Inport
  Product : Product  RndMeth=Zero
  Sum : Sum
  gain : Constant  Value=KINP_LaneC0L_m_gain
  offset : Constant  Value=KINP_LaneC0L_m_offset
  out : Outport
  wires:
  offset/1 -> Sum/2
  Product/1 -> Sum/1
  gain/1 -> Product/2
  in/1 -> Product/1
  Sum/1 -> out/1

== system inp/INP/EPM_signals/LaneC0Left/min_max_limit ==
  in : Inport
  MinMax10 : MinMax  Function=max  Inputs=2
  MinMax5 : MinMax  Inputs=2
  maxval : Constant  Value=KINP_LaneC0L_m_maxval
  minval : Constant  Value=KINP_LaneC0L_m_minval
  out : Outport
  wires:
  minval/1 -> MinMax10/2
  maxval/1 -> MinMax5/2
  MinMax10/1 -> out/1
  in/1 -> MinMax5/1
  MinMax5/1 -> MinMax10/1

== system inp/INP/EPM_signals/LaneC0Right/conv_gain_offset ==
  in : Inport
  Product : Product  RndMeth=Zero
  Sum : Sum
  gain : Constant  Value=KINP_LaneC0R_m_gain
  offset : Constant  Value=KINP_LaneC0R_m_offset
  out : Outport
  wires:
  offset/1 -> Sum/2
  Product/1 -> Sum/1
  gain/1 -> Product/2
  in/1 -> Product/1
  Sum/1 -> out/1

== system inp/INP/EPM_signals/LaneC0Right/min_max_limit ==
  in : Inport
  MinMax10 : MinMax  Function=max  Inputs=2
  MinMax5 : MinMax  Inputs=2
  maxval : Constant  Value=KINP_LaneC0R_m_maxval
  minval : Constant  Value=KINP_LaneC0R_m_minval
  out : Outport
  wires:
  minval/1 -> MinMax10/2
  maxval/1 -> MinMax5/2
  MinMax10/1 -> out/1
  in/1 -> MinMax5/1
  MinMax5/1 -> MinMax10/1

== system inp/INP/EPM_signals/LaneC1Left/conv_gain_offset ==
  in : Inport
  Product : Product  RndMeth=Zero
  Sum : Sum
  gain : Constant  Value=KINP_LaneC1L_rad_gain
  offset : Constant  Value=KINP_LaneC1L_rad_offset
  out : Outport
  wires:
  Sum/1 -> out/1
  in/1 -> Product/1
  gain/1 -> Product/2
  Product/1 -> Sum/1
  offset/1 -> Sum/2

== system inp/INP/EPM_signals/LaneC1Left/min_max_limit ==
  in : Inport
  MinMax10 : MinMax  Function=max  Inputs=2
  MinMax5 : MinMax  Inputs=2
  maxval : Constant  Value=KINP_LaneC1L_rad_maxval
  minval : Constant  Value=KINP_LaneC1L_rad_minval
  out : Outport
  wires:
  MinMax5/1 -> MinMax10/1
  in/1 -> MinMax5/1
  MinMax10/1 -> out/1
  maxval/1 -> MinMax5/2
  minval/1 -> MinMax10/2

== system inp/INP/EPM_signals/LaneC1Right/conv_gain_offset ==
  in : Inport
  Product : Product  RndMeth=Zero
  Sum : Sum
  gain : Constant  Value=KINP_LaneC1R_rad_gain
  offset : Constant  Value=KINP_LaneC1R_rad_offset
  out : Outport
  wires:
  Sum/1 -> out/1
  in/1 -> Product/1
  gain/1 -> Product/2
  Product/1 -> Sum/1
  offset/1 -> Sum/2

== system inp/INP/EPM_signals/LaneC1Right/min_max_limit ==
  in : Inport
  MinMax10 : MinMax  Function=max  Inputs=2
  MinMax5 : MinMax  Inputs=2
  maxval : Constant  Value=KINP_LaneC1R_rad_maxval
  minval : Constant  Value=KINP_LaneC1R_rad_minval
  out : Outport
  wires:
  MinMax5/1 -> MinMax10/1
  in/1 -> MinMax5/1
  MinMax10/1 -> out/1
  maxval/1 -> MinMax5/2
  minval/1 -> MinMax10/2

== system inp/INP/EPM_signals/LaneC2Left/conv_gain_offset ==
  in : Inport
  Product : Product  RndMeth=Zero
  Sum : Sum
  gain : Constant  Value=KINP_LaneC2L_rat_gain
  offset : Constant  Value=KINP_LaneC2L_rat_offset
  out : Outport
  wires:
  offset/1 -> Sum/2
  Product/1 -> Sum/1
  gain/1 -> Product/2
  in/1 -> Product/1
  Sum/1 -> out/1

== system inp/INP/EPM_signals/LaneC2Left/min_max_limit ==
  in : Inport
  MinMax10 : MinMax  Function=max  Inputs=2
  MinMax5 : MinMax  Inputs=2
  maxval : Constant  Value=KINP_LaneC2L_rat_maxval
  minval : Constant  Value=KINP_LaneC2L_rat_minval
  out : Outport
  wires:
  minval/1 -> MinMax10/2
  maxval/1 -> MinMax5/2
  MinMax10/1 -> out/1
  in/1 -> MinMax5/1
  MinMax5/1 -> MinMax10/1

== system inp/INP/EPM_signals/LaneC2Right/conv_gain_offset ==
  in : Inport
  Product : Product  RndMeth=Zero
  Sum : Sum
  gain : Constant  Value=KINP_LaneC2R_rat_gain
  offset : Constant  Value=KINP_LaneC2R_rat_offset
  out : Outport
  wires:
  offset/1 -> Sum/2
  Product/1 -> Sum/1
  gain/1 -> Product/2
  in/1 -> Product/1
  Sum/1 -> out/1

== system inp/INP/EPM_signals/LaneC2Right/min_max_limit ==
  in : Inport
  MinMax10 : MinMax  Function=max  Inputs=2
  MinMax5 : MinMax  Inputs=2
  maxval : Constant  Value=KINP_LaneC2R_rat_maxval
  minval : Constant  Value=KINP_LaneC2R_rat_minval
  out : Outport
  wires:
  minval/1 -> MinMax10/2
  maxval/1 -> MinMax5/2
  MinMax10/1 -> out/1
  in/1 -> MinMax5/1
  MinMax5/1 -> MinMax10/1

== system inp/INP/EPM_signals/LaneC3Left/conv_gain_offset ==
  in : Inport
  Product : Product  RndMeth=Zero
  Sum : Sum
  gain : Constant  Value=KINP_LaneC3L_rat_gain
  offset : Constant  Value=KINP_LaneC3L_rat_offset
  out : Outport
  wires:
  Sum/1 -> out/1
  in/1 -> Product/1
  gain/1 -> Product/2
  Product/1 -> Sum/1
  offset/1 -> Sum/2

== system inp/INP/EPM_signals/LaneC3Left/min_max_limit ==
  in : Inport
  MinMax10 : MinMax  Function=max  Inputs=2
  MinMax5 : MinMax  Inputs=2
  maxval : Constant  Value=KINP_LaneC3L_rat_maxval
  minval : Constant  Value=KINP_LaneC3L_rat_minval
  out : Outport
  wires:
  MinMax5/1 -> MinMax10/1
  in/1 -> MinMax5/1
  MinMax10/1 -> out/1
  maxval/1 -> MinMax5/2
  minval/1 -> MinMax10/2

== system inp/INP/EPM_signals/LaneC3Right/conv_gain_offset ==
  in : Inport
  Product : Product  RndMeth=Zero
  Sum : Sum
  gain : Constant  Value=KINP_LaneC3R_rat_gain
  offset : Constant  Value=KINP_LaneC3R_rat_offset
  out : Outport
  wires:
  Sum/1 -> out/1
  in/1 -> Product/1
  gain/1 -> Product/2
  Product/1 -> Sum/1
  offset/1 -> Sum/2

== system inp/INP/EPM_signals/LaneC3Right/min_max_limit ==
  in : Inport
  MinMax10 : MinMax  Function=max  Inputs=2
  MinMax5 : MinMax  Inputs=2
  maxval : Constant  Value=KINP_LaneC3R_rat_maxval
  minval : Constant  Value=KINP_LaneC3R_rat_minval
  out : Outport
  wires:
  MinMax5/1 -> MinMax10/1
  in/1 -> MinMax5/1
  MinMax10/1 -> out/1
  maxval/1 -> MinMax5/2
  minval/1 -> MinMax10/2

== system inp/INP/EPM_signals/LaneMarkWidthLeft/conv_gain_offset ==
  in : Inport
  Product : Product  RndMeth=Zero
  Sum : Sum
  gain : Constant  Value=KINP_LaneMrkWidL_m_gain
  offset : Constant  Value=KINP_LaneMrkWidL_m_offset
  out : Outport
  wires:
  Sum/1 -> out/1
  in/1 -> Product/1
  gain/1 -> Product/2
  Product/1 -> Sum/1
  offset/1 -> Sum/2
